simulink model "rfi_blk_v6_casper"  (Simulink 7.1)
settings: Solver=ode45  StartTime=0.0  StopTime=10.0  RelTol=1e-3
undefined symbols (not defined in the file): a, b
libraries: xbsIndex_r4 x13084, xps_library x4
(part 23 of 51)

== system rfi_blk_v6_casper/rfi_blk/mad_comp/Median2/Subsystem5/Median_Accumulator1 ==
  note: Provide reset to the accumulator for clearing after every window.
      | Also this can be used as an enable for calculating median.
      | An interlock logic can be created such that when all the accumulator outputs are zero, median output would be disabled (also lock it with the input enable)
  data_in : Inport  OutDataType=sfix(16)  OutScaling=2^0
  acc_reset : Inport  Port=2  OutDataType=sfix(16)  OutScaling=2^0
  Accumulator{,1..7} (8 alike) : xbsIndex_r4/Accumulator  operation=Add  n_bits=10  overflow=Wrap  scale=1  rst=on  hasbypass=off  en=on  dbl_ovrd=off  use_behavioral_HDL=on  implementation=Fabric  block_type=accum
      settings: infoedit="Adder or subtractor-based accumulator.   Output type and binary point position match the input.<P><P>Hardware notes: When \"Reinitialize with input 'b' on reset\" is selected, the accumulator is forced to run at the system rate even if the input 'b' is running at a slower rate."  block_version=10.1.3
  Constant : xbsIndex_r4/Constant  arith_type=Signed (2's comp)  const=bin_val1  n_bits=8  bin_pt=0  explicit_period=on  period=1  equ=P=C  opselect=C  inp2=PCIN>>17  opr=+  inp1=P  carry=CIN  dbl_ovrd=off  block_type=constant
  Constant1 : xbsIndex_r4/Constant  arith_type=Signed (2's comp)  const=bin_val2  n_bits=8  bin_pt=0  explicit_period=on  period=1  equ=P=C  opselect=C  inp2=PCIN>>17  opr=+  inp1=P  carry=CIN  dbl_ovrd=off  block_type=constant
  Constant2 : xbsIndex_r4/Constant  arith_type=Signed (2's comp)  const=bin_val3  n_bits=8  bin_pt=0  explicit_period=on  period=1  equ=P=C  opselect=C  inp2=PCIN>>17  opr=+  inp1=P  carry=CIN  dbl_ovrd=off  block_type=constant
  Constant3 : xbsIndex_r4/Constant  arith_type=Signed (2's comp)  const=bin_val4  n_bits=8  bin_pt=0  explicit_period=on  period=1  equ=P=C  opselect=C  inp2=PCIN>>17  opr=+  inp1=P  carry=CIN  dbl_ovrd=off  block_type=constant
  Constant4 : xbsIndex_r4/Constant  arith_type=Signed (2's comp)  const=bin_val5  n_bits=8  bin_pt=0  explicit_period=on  period=1  equ=P=C  opselect=C  inp2=PCIN>>17  opr=+  inp1=P  carry=CIN  dbl_ovrd=off  block_type=constant
  Constant5 : xbsIndex_r4/Constant  arith_type=Signed (2's comp)  const=bin_val6  n_bits=8  bin_pt=0  explicit_period=on  period=1  equ=P=C  opselect=C  inp2=PCIN>>17  opr=+  inp1=P  carry=CIN  dbl_ovrd=off  block_type=constant
  Constant6 : xbsIndex_r4/Constant  arith_type=Signed (2's comp)  const=bin_val7  n_bits=8  bin_pt=0  explicit_period=on  period=1  equ=P=C  opselect=C  inp2=PCIN>>17  opr=+  inp1=P  carry=CIN  dbl_ovrd=off  block_type=constant
  Constant7 : xbsIndex_r4/Constant  arith_type=Signed (2's comp)  const=bin_val8  n_bits=8  bin_pt=0  explicit_period=on  period=1  equ=P=C  opselect=C  inp2=PCIN>>17  opr=+  inp1=P  carry=CIN  dbl_ovrd=off  block_type=constant
  Constant8 : xbsIndex_r4/Constant  arith_type=Unsigned  const=1  n_bits=8  bin_pt=0  explicit_period=on  period=1  equ=P=C  opselect=C  inp2=PCIN>>17  opr=+  inp1=P  carry=CIN  dbl_ovrd=off  block_type=constant
  Convert{1..8} (8 alike) : xbsIndex_r4/Convert  arith_type=Boolean  n_bits=16  bin_pt=14  quantization=Truncate  overflow=Wrap  latency=0  dbl_ovrd=off  pipeline=off  block_type=convert
      settings: block_version=10.1.3
  Relational{,1..7} (8 alike) : xbsIndex_r4/Relational  mode=a<=b  en=off  latency=1  dbl_ovrd=off  block_type=relational
      settings: block_version=10.1.3
  Subsystem : Subsystem
  Subsystem1 : Subsystem
  Subsystem2 : Subsystem
  Subsystem3 : Subsystem
  Subsystem4 : Subsystem
  Subsystem5 : Subsystem
  Subsystem6 : Subsystem
  Subsystem7 : Subsystem
  Out1 : Outport
  Out2 : Outport  Port=2
  Out3 : Outport  Port=3
  Out4 : Outport  Port=4
  Out5 : Outport  Port=5
  Out6 : Outport  Port=6
  Out7 : Outport  Port=7
  Out8 : Outport  Port=8
  inc_signal : Outport  Port=9
  wires:
  Constant/1 -> Relational/2
  Constant1/1 -> Relational1/2
  Constant2/1 -> Relational2/2
  Constant4/1 -> Relational4/2
  Constant3/1 -> Relational3/2
  Constant5/1 -> Relational5/2
  Constant6/1 -> Relational6/2
  Constant7/1 -> Relational7/2
  data_in/1 -> Relational/1, Relational1/1, Relational2/1, Relational3/1, Relational4/1, Relational5/1, Relational6/1, Relational7/1
  Constant8/1 -> Accumulator/1, Accumulator1/1, Accumulator2/1, Accumulator3/1, Accumulator4/1, Accumulator5/1, Accumulator6/1, Accumulator7/1
  Relational/1 -> Convert3/1
  Relational1/1 -> Convert1/1
  Relational2/1 -> Convert2/1
  Relational3/1 -> Convert4/1
  Relational4/1 -> Convert5/1
  Relational5/1 -> Convert6/1
  Relational6/1 -> Convert7/1
  Relational7/1 -> Convert8/1
  Convert3/1 -> Accumulator/3
  Convert1/1 -> Accumulator1/3
  Convert2/1 -> Accumulator2/3
  Convert4/1 -> Accumulator3/3
  Convert5/1 -> Accumulator4/3
  Convert6/1 -> Accumulator5/3
  Convert7/1 -> Accumulator6/3
  Convert8/1 -> Accumulator7/3, inc_signal/1
  acc_reset/1 -> Accumulator/2, Accumulator1/2, Accumulator2/2, Accumulator3/2, Accumulator4/2, Accumulator5/2, Accumulator6/2, Accumulator7/2, Subsystem/2(acc_reset_in), Subsystem1/2(acc_reset_in), Subsystem2/2(acc_reset_in), Subsystem3/2(acc_reset_in), Subsystem4/2(acc_reset_in), Subsystem5/2(acc_reset_in), Subsystem6/2(acc_reset_in), Subsystem7/2(acc_reset_in)
  Accumulator/1 -> Subsystem/1(acc_out_in)
  Subsystem/1(adder_out) -> Out1/1
  Accumulator1/1 -> Subsystem1/1(acc_out_in)
  Subsystem1/1(adder_out) -> Out2/1
  Accumulator2/1 -> Subsystem2/1(acc_out_in)
  Subsystem2/1(adder_out) -> Out3/1
  Subsystem3/1(adder_out) -> Out4/1
  Accumulator3/1 -> Subsystem3/1(acc_out_in)
  Accumulator4/1 -> Subsystem4/1(acc_out_in)
  Subsystem4/1(adder_out) -> Out5/1
  Accumulator5/1 -> Subsystem5/1(acc_out_in)
  Subsystem5/1(adder_out) -> Out6/1
  Subsystem6/1(adder_out) -> Out7/1
  Accumulator6/1 -> Subsystem6/1(acc_out_in)
  Accumulator7/1 -> Subsystem7/1(acc_out_in)
  Subsystem7/1(adder_out) -> Out8/1

== system rfi_blk_v6_casper/rfi_blk/mad_comp/Median2/Subsystem5/Median_Accumulator2 ==
  data_in : Inport  OutDataType=sfix(16)  OutScaling=2^0
  acc_reset : Inport  Port=2  OutDataType=sfix(16)  OutScaling=2^0
  Accumulator{,1..7} (8 alike) : xbsIndex_r4/Accumulator  operation=Add  n_bits=10  overflow=Wrap  scale=1  rst=on  hasbypass=off  en=on  dbl_ovrd=off  use_behavioral_HDL=on  implementation=Fabric  block_type=accum
      settings: infoedit="Adder or subtractor-based accumulator.   Output type and binary point position match the input.<P><P>Hardware notes: When \"Reinitialize with input 'b' on reset\" is selected, the accumulator is forced to run at the system rate even if the input 'b' is running at a slower rate."  block_version=10.1.3
  Constant : xbsIndex_r4/Constant  arith_type=Signed (2's comp)  const=bin_val9  n_bits=8  bin_pt=0  explicit_period=on  period=1  equ=P=C  opselect=C  inp2=PCIN>>17  opr=+  inp1=P  carry=CIN  dbl_ovrd=off  block_type=constant
  Constant1 : xbsIndex_r4/Constant  arith_type=Signed (2's comp)  const=bin_val10  n_bits=8  bin_pt=0  explicit_period=on  period=1  equ=P=C  opselect=C  inp2=PCIN>>17  opr=+  inp1=P  carry=CIN  dbl_ovrd=off  block_type=constant
  Constant2 : xbsIndex_r4/Constant  arith_type=Signed (2's comp)  const=bin_val11  n_bits=8  bin_pt=0  explicit_period=on  period=1  equ=P=C  opselect=C  inp2=PCIN>>17  opr=+  inp1=P  carry=CIN  dbl_ovrd=off  block_type=constant
  Constant3 : xbsIndex_r4/Constant  arith_type=Signed (2's comp)  const=bin_val12  n_bits=8  bin_pt=0  explicit_period=on  period=1  equ=P=C  opselect=C  inp2=PCIN>>17  opr=+  inp1=P  carry=CIN  dbl_ovrd=off  block_type=constant
  Constant4 : xbsIndex_r4/Constant  arith_type=Signed (2's comp)  const=bin_val13  n_bits=8  bin_pt=0  explicit_period=on  period=1  equ=P=C  opselect=C  inp2=PCIN>>17  opr=+  inp1=P  carry=CIN  dbl_ovrd=off  block_type=constant
  Constant5 : xbsIndex_r4/Constant  arith_type=Signed (2's comp)  const=bin_val14  n_bits=8  bin_pt=0  explicit_period=on  period=1  equ=P=C  opselect=C  inp2=PCIN>>17  opr=+  inp1=P  carry=CIN  dbl_ovrd=off  block_type=constant
  Constant6 : xbsIndex_r4/Constant  arith_type=Signed (2's comp)  const=bin_val15  n_bits=8  bin_pt=0  explicit_period=on  period=1  equ=P=C  opselect=C  inp2=PCIN>>17  opr=+  inp1=P  carry=CIN  dbl_ovrd=off  block_type=constant
  Constant7 : xbsIndex_r4/Constant  arith_type=Signed (2's comp)  const=bin_val16  n_bits=8  bin_pt=0  explicit_period=on  period=1  equ=P=C  opselect=C  inp2=PCIN>>17  opr=+  inp1=P  carry=CIN  dbl_ovrd=off  block_type=constant
  Constant8 : xbsIndex_r4/Constant  arith_type=Unsigned  const=1  n_bits=8  bin_pt=0  explicit_period=on  period=1  equ=P=C  opselect=C  inp2=PCIN>>17  opr=+  inp1=P  carry=CIN  dbl_ovrd=off  block_type=constant
  Convert{1..8} (8 alike) : xbsIndex_r4/Convert  arith_type=Boolean  n_bits=16  bin_pt=14  quantization=Truncate  overflow=Wrap  latency=0  dbl_ovrd=off  pipeline=off  block_type=convert
      settings: block_version=10.1.3
  Relational{,1..7} (8 alike) : xbsIndex_r4/Relational  mode=a<=b  en=off  latency=1  dbl_ovrd=off  block_type=relational
      settings: block_version=10.1.3
  Subsystem1 : Subsystem
  Subsystem2 : Subsystem
  Subsystem3 : Subsystem
  Subsystem4 : Subsystem
  Subsystem5 : Subsystem
  Subsystem6 : Subsystem
  Subsystem7 : Subsystem
  Subsystem8 : Subsystem
  Out1 : Outport
  Out2 : Outport  Port=2
  Out3 : Outport  Port=3
  Out4 : Outport  Port=4
  Out5 : Outport  Port=5
  Out6 : Outport  Port=6
  Out7 : Outport  Port=7
  Out8 : Outport  Port=8
  inc_signal : Outport  Port=9
  wires:
  Convert8/1 -> Accumulator7/3
  Convert7/1 -> Accumulator6/3
  Convert6/1 -> Accumulator5/3
  Convert5/1 -> Accumulator4/3
  Convert4/1 -> Accumulator3/3
  Convert2/1 -> Accumulator2/3
  Convert1/1 -> Accumulator1/3
  Convert3/1 -> Accumulator/3
  Relational7/1 -> inc_signal/1, Convert8/1
  Relational6/1 -> Convert7/1
  Relational5/1 -> Convert6/1
  Relational4/1 -> Convert5/1
  Relational3/1 -> Convert4/1
  Relational2/1 -> Convert2/1
  Relational1/1 -> Convert1/1
  Constant8/1 -> Accumulator7/1, Accumulator6/1, Accumulator5/1, Accumulator4/1, Accumulator3/1, Accumulator2/1, Accumulator1/1, Accumulator/1
  acc_reset/1 -> Accumulator5/2, Accumulator6/2, Accumulator7/2, Accumulator4/2, Accumulator3/2, Accumulator2/2, Accumulator1/2, Accumulator/2, Subsystem2/2(acc_reset_in), Subsystem3/2(acc_reset_in), Subsystem4/2(acc_reset_in), Subsystem5/2(acc_reset_in), Subsystem6/2(acc_reset_in), Subsystem7/2(acc_reset_in), Subsystem8/2(acc_reset_in), Subsystem1/2(acc_reset_in)
  data_in/1 -> Relational7/1, Relational6/1, Relational5/1, Relational4/1, Relational3/1, Relational2/1, Relational1/1, Relational/1
  Constant7/1 -> Relational7/2
  Constant6/1 -> Relational6/2
  Constant5/1 -> Relational5/2
  Constant3/1 -> Relational3/2
  Constant4/1 -> Relational4/2
  Constant2/1 -> Relational2/2
  Constant1/1 -> Relational1/2
  Constant/1 -> Relational/2
  Relational/1 -> Convert3/1
  Subsystem2/1(adder_out) -> Out1/1
  Accumulator/1 -> Subsystem2/1(acc_out_in)
  Accumulator1/1 -> Subsystem1/1(acc_out_in)
  Subsystem1/1(adder_out) -> Out2/1
  Accumulator2/1 -> Subsystem3/1(acc_out_in)
  Subsystem3/1(adder_out) -> Out3/1
  Subsystem4/1(adder_out) -> Out4/1
  Accumulator3/1 -> Subsystem4/1(acc_out_in)
  Subsystem5/1(adder_out) -> Out5/1
  Accumulator4/1 -> Subsystem5/1(acc_out_in)
  Subsystem6/1(adder_out) -> Out6/1
  Accumulator5/1 -> Subsystem6/1(acc_out_in)
  Subsystem7/1(adder_out) -> Out7/1
  Accumulator6/1 -> Subsystem7/1(acc_out_in)
  Subsystem8/1(adder_out) -> Out8/1
  Accumulator7/1 -> Subsystem8/1(acc_out_in)

== system rfi_blk_v6_casper/rfi_blk/mad_comp/Median2/Subsystem5/Median_Location1 ==
  In1 : Inport  OutDataType=sfix(16)  OutScaling=2^0
  In2 : Inport  Port=2  OutDataType=sfix(16)  OutScaling=2^0
  In3 : Inport  Port=3  OutDataType=sfix(16)  OutScaling=2^0
  In4 : Inport  Port=4  OutDataType=sfix(16)  OutScaling=2^0
  In5 : Inport  Port=5  OutDataType=sfix(16)  OutScaling=2^0
  In6 : Inport  Port=6  OutDataType=sfix(16)  OutScaling=2^0
  In7 : Inport  Port=7  OutDataType=sfix(16)  OutScaling=2^0
  In8 : Inport  Port=8  OutDataType=sfix(16)  OutScaling=2^0
  median_loc : Inport  Port=9  OutDataType=sfix(16)  OutScaling=2^0
  Constant{10,12,14,16,18,20,22,24} (8 alike) : xbsIndex_r4/Constant  arith_type=Boolean  const=1  n_bits=1  bin_pt=0  explicit_period=on  period=1  equ=P=C  opselect=C  inp2=PCIN>>17  opr=+  inp1=P  carry=CIN  dbl_ovrd=off  block_type=constant
  Constant{9,11,13,15,17,19,21,23} (8 alike) : xbsIndex_r4/Constant  arith_type=Boolean  const=0  n_bits=1  bin_pt=0  explicit_period=on  period=1  equ=P=C  opselect=C  inp2=PCIN>>17  opr=+  inp1=P  carry=CIN  dbl_ovrd=off  block_type=constant
  Constant25 : xbsIndex_r4/Constant  arith_type=Signed (2's comp)  const=0  n_bits=8  bin_pt=0  explicit_period=on  period=1  equ=P=C  opselect=C  inp2=PCIN>>17  opr=+  inp1=P  carry=CIN  dbl_ovrd=off  block_type=constant
  Constant26 : xbsIndex_r4/Constant  arith_type=Signed (2's comp)  const=bin_val1  n_bits=8  bin_pt=0  explicit_period=on  period=1  equ=P=C  opselect=C  inp2=PCIN>>17  opr=+  inp1=P  carry=CIN  dbl_ovrd=off  block_type=constant
  Constant27 : xbsIndex_r4/Constant  arith_type=Signed (2's comp)  const=bin_val2  n_bits=8  bin_pt=0  explicit_period=on  period=1  equ=P=C  opselect=C  inp2=PCIN>>17  opr=+  inp1=P  carry=CIN  dbl_ovrd=off  block_type=constant
  Constant28 : xbsIndex_r4/Constant  arith_type=Signed (2's comp)  const=bin_val3  n_bits=8  bin_pt=0  explicit_period=on  period=1  equ=P=C  opselect=C  inp2=PCIN>>17  opr=+  inp1=P  carry=CIN  dbl_ovrd=off  block_type=constant
  Constant29 : xbsIndex_r4/Constant  arith_type=Signed (2's comp)  const=bin_val4  n_bits=8  bin_pt=0  explicit_period=on  period=1  equ=P=C  opselect=C  inp2=PCIN>>17  opr=+  inp1=P  carry=CIN  dbl_ovrd=off  block_type=constant
  Constant30 : xbsIndex_r4/Constant  arith_type=Signed (2's comp)  const=bin_val5  n_bits=8  bin_pt=0  explicit_period=on  period=1  equ=P=C  opselect=C  inp2=PCIN>>17  opr=+  inp1=P  carry=CIN  dbl_ovrd=off  block_type=constant
  Constant31 : xbsIndex_r4/Constant  arith_type=Signed (2's comp)  const=bin_val6  n_bits=8  bin_pt=0  explicit_period=on  period=1  equ=P=C  opselect=C  inp2=PCIN>>17  opr=+  inp1=P  carry=CIN  dbl_ovrd=off  block_type=constant
  Constant32 : xbsIndex_r4/Constant  arith_type=Signed (2's comp)  const=bin_val7  n_bits=8  bin_pt=0  explicit_period=on  period=1  equ=P=C  opselect=C  inp2=PCIN>>17  opr=+  inp1=P  carry=CIN  dbl_ovrd=off  block_type=constant
  Constant33 : xbsIndex_r4/Constant  arith_type=Signed (2's comp)  const=bin_val8  n_bits=8  bin_pt=0  explicit_period=on  period=1  equ=P=C  opselect=C  inp2=PCIN>>17  opr=+  inp1=P  carry=CIN  dbl_ovrd=off  block_type=constant
  Convert : xbsIndex_r4/Convert  arith_type=Boolean  n_bits=16  bin_pt=14  quantization=Truncate  overflow=Wrap  latency=0  dbl_ovrd=off  pipeline=off  block_type=convert
      settings: block_version=10.1.3
  Inverter{,1..6} (7 alike) : xbsIndex_r4/Inverter  en=off  latency=0  dbl_ovrd=off  block_type=inv
  Logical{,2..3,5,7,9,11} (7 alike) : xbsIndex_r4/Logical  logical_function=AND  inputs=2  en=off  latency=0  precision=Full  arith_type=Unsigned  n_bits=16  bin_pt=0  align_bp=on  dbl_ovrd=off  block_type=logical
  Logical1 : xbsIndex_r4/Logical  logical_function=OR  inputs=8  en=off  latency=1  precision=User Defined  arith_type=Unsigned  n_bits=1  bin_pt=0  align_bp=on  dbl_ovrd=off  block_type=logical
  Logical13 : xbsIndex_r4/Logical  logical_function=OR  inputs=8  en=off  latency=1  precision=User Defined  arith_type="Signed  (2's comp)"  n_bits=8  bin_pt=0  align_bp=on  dbl_ovrd=off  block_type=logical
  Mux{,1..7} (8 alike) : xbsIndex_r4/Mux  inputs=2  en=off  latency=0  precision=Full  arith_type="Signed  (2's comp)"  n_bits=8  bin_pt=0  quantization=Truncate  overflow=Wrap  dbl_ovrd=off  block_type=mux
  Mux{8..15} (8 alike) : xbsIndex_r4/Mux  inputs=2  en=off  latency=0  precision=User Defined  arith_type="Signed  (2's comp)"  n_bits=8  bin_pt=0  quantization=Truncate  overflow=Wrap  dbl_ovrd=off  block_type=mux
  Relational{1..8} (8 alike) : xbsIndex_r4/Relational  mode=a>=b  en=off  latency=1  dbl_ovrd=off  block_type=relational
      settings: block_version=10.1.3
  Scope1 : Scope  NumInputPorts=8
      settings: YMin=-5~-5~-5~-5~-5~-5~-5~-5  YMax=5~5~5~5~5~5~5~5  SaveName=ScopeData2
  Scope2 : Scope  NumInputPorts=2
      settings: YMin=-5~-5  YMax=5~5  SaveName=ScopeData3
  Scope3 : Scope  NumInputPorts=2
      settings: YMin=-5~-5  YMax=5~5  SaveName=ScopeData4
  Scope5 : Scope  NumInputPorts=2
      settings: YMin=-5~-5  YMax=5~5  SaveName=ScopeData16
  Out1 : Outport  OutDataType=sfix(16)  OutScaling=2^0
  sel1 : Outport  Port=2  OutDataType=sfix(16)  OutScaling=2^0
  wires:
  median_loc/1 -> Relational1/2, Relational2/2, Relational3/2, Relational4/2, Relational5/2, Relational6/2, Relational7/2, Relational8/2
  Constant9/1 -> Mux/2
  Constant10/1 -> Mux/3
  Relational1/1 -> Mux/1, Inverter/1
  Mux/1 -> [A]
  Inverter/1 -> Logical/1
  Relational2/1 -> Logical/2, Inverter1/1
  Logical/1 -> Mux1/1
  Constant11/1 -> Mux1/2
  Constant12/1 -> Mux1/3
  Relational3/1 -> Logical2/2, Inverter2/1, Scope5/1
  Logical2/1 -> Mux2/1
  Constant13/1 -> Mux2/2
  Constant14/1 -> Mux2/3
  Inverter2/1 -> Logical3/1
  Logical3/1 -> Mux3/1
  Constant15/1 -> Mux3/2
  Constant16/1 -> Mux3/3
  Relational5/1 -> Logical5/2, Inverter4/1, Scope5/2
  Logical5/1 -> Mux4/1
  Constant17/1 -> Mux4/2
  Constant18/1 -> Mux4/3
  Relational6/1 -> Logical7/2, Inverter5/1
  Logical7/1 -> Mux5/1
  Constant19/1 -> Mux5/2
  Constant20/1 -> Mux5/3
  Inverter5/1 -> Logical9/1
  Relational7/1 -> Logical9/2, Inverter6/1
  Constant21/1 -> Mux6/2
  Constant22/1 -> Mux6/3
  Logical9/1 -> Mux6/1
  Inverter6/1 -> Logical11/1
  Relational8/1 -> Logical11/2, Scope3/1
  Constant23/1 -> Mux7/2
  Constant24/1 -> Mux7/3
  Logical11/1 -> Mux7/1
  Mux7/1 -> [H]
  Constant25/1 -> Mux8/2, Mux9/2, Mux10/2, Mux11/2, Mux12/2, Mux13/2, Mux14/2, Mux15/2
  Constant26/1 -> Mux8/3
  Constant27/1 -> Mux9/3
  Constant28/1 -> Mux10/3
  Constant29/1 -> Mux11/3
  Constant30/1 -> Mux12/3
  Constant31/1 -> Mux13/3
  Constant32/1 -> Mux14/3
  Constant33/1 -> Mux15/3
  [A] -> Mux8/1
  [B] -> Mux9/1
  [C] -> Mux10/1
  [D] -> Mux11/1
  [E] -> Mux12/1
  [F] -> Mux13/1
  [G] -> Mux14/1
  [H] -> Mux15/1
  Mux8/1 -> Logical13/1, Scope1/1
  Mux9/1 -> Logical13/2, Scope1/2
  Mux10/1 -> Logical13/3, Scope1/3
  Mux11/1 -> Logical13/4, Scope1/4
  Mux12/1 -> Logical13/5, Scope1/5
  Mux13/1 -> Logical13/6, Scope1/6
  Mux14/1 -> Logical13/7, Scope1/7
  Mux15/1 -> Logical13/8, Scope1/8
  Logical13/1 -> Out1/1
  In1/1 -> Relational1/1
  In2/1 -> Relational2/1
  In3/1 -> Relational3/1
  In4/1 -> Relational4/1
  In5/1 -> Relational5/1
  In6/1 -> Relational6/1
  In7/1 -> Relational7/1
  In8/1 -> Relational8/1, Scope3/2
  Mux1/1 -> [B]
  Mux2/1 -> [C]
  Mux3/1 -> [D]
  Mux4/1 -> [E]
  Mux5/1 -> [F]
  Mux6/1 -> [G]
  Inverter1/1 -> Logical2/1
  Relational4/1 -> Logical3/2, Inverter3/1
  Inverter3/1 -> Logical5/1
  Inverter4/1 -> Logical7/1
  [A] -> Logical1/1
  [B] -> Logical1/2
  [C] -> Logical1/3
  [D] -> Logical1/4
  [E] -> Logical1/5
  [F] -> Logical1/6
  [G] -> Logical1/7
  [H] -> Logical1/8
  Logical1/1 -> Convert/1
  Convert/1 -> sel1/1

== system rfi_blk_v6_casper/rfi_blk/mad_comp/Median2/Subsystem5/Median_Location2 ==
  In1 : Inport  OutDataType=sfix(16)  OutScaling=2^0
  In2 : Inport  Port=2  OutDataType=sfix(16)  OutScaling=2^0
  In3 : Inport  Port=3  OutDataType=sfix(16)  OutScaling=2^0
  In4 : Inport  Port=4  OutDataType=sfix(16)  OutScaling=2^0
  In5 : Inport  Port=5  OutDataType=sfix(16)  OutScaling=2^0
  In6 : Inport  Port=6  OutDataType=sfix(16)  OutScaling=2^0
  In7 : Inport  Port=7  OutDataType=sfix(16)  OutScaling=2^0
  In8 : Inport  Port=8  OutDataType=sfix(16)  OutScaling=2^0
  median_loc : Inport  Port=9  OutDataType=sfix(16)  OutScaling=2^0
  Constant{10,12,14,16,18,20,22,24} (8 alike) : xbsIndex_r4/Constant  arith_type=Boolean  const=1  n_bits=1  bin_pt=0  explicit_period=on  period=1  equ=P=C  opselect=C  inp2=PCIN>>17  opr=+  inp1=P  carry=CIN  dbl_ovrd=off  block_type=constant
  Constant{9,11,13,15,17,19,21,23} (8 alike) : xbsIndex_r4/Constant  arith_type=Boolean  const=0  n_bits=1  bin_pt=0  explicit_period=on  period=1  equ=P=C  opselect=C  inp2=PCIN>>17  opr=+  inp1=P  carry=CIN  dbl_ovrd=off  block_type=constant
  Constant25 : xbsIndex_r4/Constant  arith_type=Signed (2's comp)  const=0  n_bits=8  bin_pt=0  explicit_period=on  period=1  equ=P=C  opselect=C  inp2=PCIN>>17  opr=+  inp1=P  carry=CIN  dbl_ovrd=off  block_type=constant
  Constant26 : xbsIndex_r4/Constant  arith_type=Signed (2's comp)  const=bin_val9  n_bits=8  bin_pt=0  explicit_period=on  period=1  equ=P=C  opselect=C  inp2=PCIN>>17  opr=+  inp1=P  carry=CIN  dbl_ovrd=off  block_type=constant
  Constant27 : xbsIndex_r4/Constant  arith_type=Signed (2's comp)  const=bin_val10  n_bits=8  bin_pt=0  explicit_period=on  period=1  equ=P=C  opselect=C  inp2=PCIN>>17  opr=+  inp1=P  carry=CIN  dbl_ovrd=off  block_type=constant
  Constant28 : xbsIndex_r4/Constant  arith_type=Signed (2's comp)  const=bin_val11  n_bits=8  bin_pt=0  explicit_period=on  period=1  equ=P=C  opselect=C  inp2=PCIN>>17  opr=+  inp1=P  carry=CIN  dbl_ovrd=off  block_type=constant
  Constant29 : xbsIndex_r4/Constant  arith_type=Signed (2's comp)  const=bin_val12  n_bits=8  bin_pt=0  explicit_period=on  period=1  equ=P=C  opselect=C  inp2=PCIN>>17  opr=+  inp1=P  carry=CIN  dbl_ovrd=off  block_type=constant
  Constant30 : xbsIndex_r4/Constant  arith_type=Signed (2's comp)  const=bin_val13  n_bits=8  bin_pt=0  explicit_period=on  period=1  equ=P=C  opselect=C  inp2=PCIN>>17  opr=+  inp1=P  carry=CIN  dbl_ovrd=off  block_type=constant
  Constant31 : xbsIndex_r4/Constant  arith_type=Signed (2's comp)  const=bin_val14  n_bits=8  bin_pt=0  explicit_period=on  period=1  equ=P=C  opselect=C  inp2=PCIN>>17  opr=+  inp1=P  carry=CIN  dbl_ovrd=off  block_type=constant
  Constant32 : xbsIndex_r4/Constant  arith_type=Signed (2's comp)  const=bin_val15  n_bits=8  bin_pt=0  explicit_period=on  period=1  equ=P=C  opselect=C  inp2=PCIN>>17  opr=+  inp1=P  carry=CIN  dbl_ovrd=off  block_type=constant
  Constant33 : xbsIndex_r4/Constant  arith_type=Signed (2's comp)  const=bin_val16  n_bits=8  bin_pt=0  explicit_period=on  period=1  equ=P=C  opselect=C  inp2=PCIN>>17  opr=+  inp1=P  carry=CIN  dbl_ovrd=off  block_type=constant
  Convert{,1..8} (9 alike) : xbsIndex_r4/Convert  arith_type=Boolean  n_bits=16  bin_pt=14  quantization=Truncate  overflow=Wrap  latency=0  dbl_ovrd=off  pipeline=off  block_type=convert
      settings: block_version=10.1.3
  Inverter{,1..6} (7 alike) : xbsIndex_r4/Inverter  en=off  latency=0  dbl_ovrd=off  block_type=inv
  Logical{,2..3,5,7,9,11} (7 alike) : xbsIndex_r4/Logical  logical_function=AND  inputs=2  en=off  latency=0  precision=Full  arith_type=Unsigned  n_bits=16  bin_pt=0  align_bp=on  dbl_ovrd=off  block_type=logical
  Logical1 : xbsIndex_r4/Logical  logical_function=OR  inputs=8  en=off  latency=1  precision=User Defined  arith_type=Unsigned  n_bits=1  bin_pt=0  align_bp=on  dbl_ovrd=off  block_type=logical
  Logical13 : xbsIndex_r4/Logical  logical_function=OR  inputs=8  en=off  latency=1  precision=User Defined  arith_type="Signed  (2's comp)"  n_bits=8  bin_pt=0  align_bp=on  dbl_ovrd=off  block_type=logical
  Mux{,1..7} (8 alike) : xbsIndex_r4/Mux  inputs=2  en=off  latency=0  precision=Full  arith_type=Unsigned  n_bits=16  bin_pt=14  quantization=Truncate  overflow=Wrap  dbl_ovrd=off  block_type=mux
  Mux{8..15} (8 alike) : xbsIndex_r4/Mux  inputs=2  en=off  latency=0  precision=User Defined  arith_type="Signed  (2's comp)"  n_bits=8  bin_pt=0  quantization=Truncate  overflow=Wrap  dbl_ovrd=off  block_type=mux
  Relational{1..8} (8 alike) : xbsIndex_r4/Relational  mode=a>=b  en=off  latency=1  dbl_ovrd=off  block_type=relational
      settings: block_version=10.1.3
  Scope1 : Scope  NumInputPorts=8
      settings: YMin=-5~-5~-5~-5~-5~-5~-5~-5  YMax=5~5~5~5~5~5~5~5  SaveName=ScopeData2
  Out3 : Outport  OutDataType=sfix(16)  OutScaling=2^0
  sel2 : Outport  Port=2  OutDataType=sfix(16)  OutScaling=2^0
  wires:
  Inverter4/1 -> Logical7/1
  Inverter3/1 -> Logical5/1
  Relational4/1 -> Inverter3/1, Logical3/2
  Inverter1/1 -> Logical2/1
  Mux6/1 -> [G]
  Mux5/1 -> [F]
  Mux4/1 -> [E]
  Mux3/1 -> [D]
  Mux2/1 -> [C]
  Mux1/1 -> [B]
  In8/1 -> Relational8/1
  In7/1 -> Relational7/1
  In6/1 -> Relational6/1
  In5/1 -> Relational5/1
  In4/1 -> Relational4/1
  In3/1 -> Relational3/1
  In2/1 -> Relational2/1
  In1/1 -> Relational1/1
  Mux15/1 -> Scope1/8, Logical13/8
  Mux14/1 -> Scope1/7, Logical13/7
  Mux13/1 -> Scope1/6, Logical13/6
  Mux12/1 -> Scope1/5, Logical13/5
  Mux11/1 -> Scope1/4, Logical13/4
  Mux10/1 -> Scope1/3, Logical13/3
  Mux9/1 -> Scope1/2, Logical13/2
  Mux8/1 -> Scope1/1, Logical13/1
  [H] -> Mux15/1
  [G] -> Mux14/1
  [F] -> Mux13/1
  [E] -> Mux12/1
  [D] -> Mux11/1
  [C] -> Mux10/1
  [B] -> Mux9/1
  [A] -> Mux8/1
  Constant33/1 -> Mux15/3
  Constant32/1 -> Mux14/3
  Constant31/1 -> Mux13/3
  Constant30/1 -> Mux12/3
  Constant29/1 -> Mux11/3
  Constant28/1 -> Mux10/3
  Constant27/1 -> Mux9/3
  Constant26/1 -> Mux8/3
  Constant25/1 -> Mux15/2, Mux14/2, Mux13/2, Mux12/2, Mux11/2, Mux10/2, Mux9/2, Mux8/2
  Mux7/1 -> [H]
  Logical11/1 -> Mux7/1
  Constant24/1 -> Mux7/3
  Constant23/1 -> Mux7/2
  Relational8/1 -> Logical11/2
  Inverter6/1 -> Logical11/1
  Logical9/1 -> Mux6/1
  Constant22/1 -> Mux6/3
  Constant21/1 -> Mux6/2
  Relational7/1 -> Inverter6/1, Logical9/2
  Inverter5/1 -> Logical9/1
  Constant20/1 -> Mux5/3
  Constant19/1 -> Mux5/2
  Logical7/1 -> Mux5/1
  Relational6/1 -> Inverter5/1, Logical7/2
  Constant18/1 -> Mux4/3
  Constant17/1 -> Mux4/2
  Logical5/1 -> Mux4/1
  Relational5/1 -> Inverter4/1, Logical5/2
  Constant16/1 -> Mux3/3
  Constant15/1 -> Mux3/2
  Logical3/1 -> Mux3/1
  Inverter2/1 -> Logical3/1
  Constant14/1 -> Mux2/3
  Constant13/1 -> Mux2/2
  Logical2/1 -> Mux2/1
  Relational3/1 -> Inverter2/1, Logical2/2
  Constant12/1 -> Mux1/3
  Constant11/1 -> Mux1/2
  Logical/1 -> Mux1/1
  Relational2/1 -> Inverter1/1, Logical/2
  Inverter/1 -> Logical/1
  Mux/1 -> [A]
  Relational1/1 -> Inverter/1, Mux/1
  Constant10/1 -> Mux/3
  Constant9/1 -> Mux/2
  median_loc/1 -> Relational8/2, Relational7/2, Relational6/2, Relational5/2, Relational4/2, Relational3/2, Relational2/2, Relational1/2
  [A] -> Convert1/1
  [B] -> Convert2/1
  [C] -> Convert3/1
  [D] -> Convert4/1
  [E] -> Convert5/1
  [F] -> Convert6/1
  [G] -> Convert7/1
  [H] -> Convert8/1
  Logical1/1 -> Convert/1
  Convert/1 -> sel2/1
  Convert1/1 -> Logical1/1
  Convert2/1 -> Logical1/2
  Convert3/1 -> Logical1/3
  Convert4/1 -> Logical1/4
  Convert5/1 -> Logical1/5
  Convert6/1 -> Logical1/6
  Convert7/1 -> Logical1/7
  Convert8/1 -> Logical1/8
  Logical13/1 -> Out3/1

== system rfi_blk_v6_casper/rfi_blk/mad_comp/Median2/Subsystem6/Median_Accumulator1 ==
  note: Provide reset to the accumulator for clearing after every window.
      | Also this can be used as an enable for calculating median.
      | An interlock logic can be created such that when all the accumulator outputs are zero, median output would be disabled (also lock it with the input enable)
  data_in : Inport  OutDataType=sfix(16)  OutScaling=2^0
  acc_reset : Inport  Port=2  OutDataType=sfix(16)  OutScaling=2^0
  Accumulator{,1..7} (8 alike) : xbsIndex_r4/Accumulator  operation=Add  n_bits=10  overflow=Wrap  scale=1  rst=on  hasbypass=off  en=on  dbl_ovrd=off  use_behavioral_HDL=on  implementation=Fabric  block_type=accum
      settings: infoedit="Adder or subtractor-based accumulator.   Output type and binary point position match the input.<P><P>Hardware notes: When \"Reinitialize with input 'b' on reset\" is selected, the accumulator is forced to run at the system rate even if the input 'b' is running at a slower rate."  block_version=10.1.3
  Constant : xbsIndex_r4/Constant  arith_type=Signed (2's comp)  const=bin_val1  n_bits=8  bin_pt=0  explicit_period=on  period=1  equ=P=C  opselect=C  inp2=PCIN>>17  opr=+  inp1=P  carry=CIN  dbl_ovrd=off  block_type=constant
  Constant1 : xbsIndex_r4/Constant  arith_type=Signed (2's comp)  const=bin_val2  n_bits=8  bin_pt=0  explicit_period=on  period=1  equ=P=C  opselect=C  inp2=PCIN>>17  opr=+  inp1=P  carry=CIN  dbl_ovrd=off  block_type=constant
  Constant2 : xbsIndex_r4/Constant  arith_type=Signed (2's comp)  const=bin_val3  n_bits=8  bin_pt=0  explicit_period=on  period=1  equ=P=C  opselect=C  inp2=PCIN>>17  opr=+  inp1=P  carry=CIN  dbl_ovrd=off  block_type=constant
  Constant3 : xbsIndex_r4/Constant  arith_type=Signed (2's comp)  const=bin_val4  n_bits=8  bin_pt=0  explicit_period=on  period=1  equ=P=C  opselect=C  inp2=PCIN>>17  opr=+  inp1=P  carry=CIN  dbl_ovrd=off  block_type=constant
  Constant4 : xbsIndex_r4/Constant  arith_type=Signed (2's comp)  const=bin_val5  n_bits=8  bin_pt=0  explicit_period=on  period=1  equ=P=C  opselect=C  inp2=PCIN>>17  opr=+  inp1=P  carry=CIN  dbl_ovrd=off  block_type=constant
  Constant5 : xbsIndex_r4/Constant  arith_type=Signed (2's comp)  const=bin_val6  n_bits=8  bin_pt=0  explicit_period=on  period=1  equ=P=C  opselect=C  inp2=PCIN>>17  opr=+  inp1=P  carry=CIN  dbl_ovrd=off  block_type=constant
  Constant6 : xbsIndex_r4/Constant  arith_type=Signed (2's comp)  const=bin_val7  n_bits=8  bin_pt=0  explicit_period=on  period=1  equ=P=C  opselect=C  inp2=PCIN>>17  opr=+  inp1=P  carry=CIN  dbl_ovrd=off  block_type=constant
  Constant7 : xbsIndex_r4/Constant  arith_type=Signed (2's comp)  const=bin_val8  n_bits=8  bin_pt=0  explicit_period=on  period=1  equ=P=C  opselect=C  inp2=PCIN>>17  opr=+  inp1=P  carry=CIN  dbl_ovrd=off  block_type=constant
  Constant8 : xbsIndex_r4/Constant  arith_type=Unsigned  const=1  n_bits=8  bin_pt=0  explicit_period=on  period=1  equ=P=C  opselect=C  inp2=PCIN>>17  opr=+  inp1=P  carry=CIN  dbl_ovrd=off  block_type=constant
  Convert{1..8} (8 alike) : xbsIndex_r4/Convert  arith_type=Boolean  n_bits=16  bin_pt=14  quantization=Truncate  overflow=Wrap  latency=0  dbl_ovrd=off  pipeline=off  block_type=convert
      settings: block_version=10.1.3
  Relational{,1..7} (8 alike) : xbsIndex_r4/Relational  mode=a<=b  en=off  latency=1  dbl_ovrd=off  block_type=relational
      settings: block_version=10.1.3
  Subsystem : Subsystem
  Subsystem1 : Subsystem
  Subsystem2 : Subsystem
  Subsystem3 : Subsystem
  Subsystem4 : Subsystem
  Subsystem5 : Subsystem
  Subsystem6 : Subsystem
  Subsystem7 : Subsystem
  Out1 : Outport
  Out2 : Outport  Port=2
  Out3 : Outport  Port=3
  Out4 : Outport  Port=4
  Out5 : Outport  Port=5
  Out6 : Outport  Port=6
  Out7 : Outport  Port=7
  Out8 : Outport  Port=8
  inc_signal : Outport  Port=9
  wires:
  Constant/1 -> Relational/2
  Constant1/1 -> Relational1/2
  Constant2/1 -> Relational2/2
  Constant4/1 -> Relational4/2
  Constant3/1 -> Relational3/2
  Constant5/1 -> Relational5/2
  Constant6/1 -> Relational6/2
  Constant7/1 -> Relational7/2
  data_in/1 -> Relational/1, Relational1/1, Relational2/1, Relational3/1, Relational4/1, Relational5/1, Relational6/1, Relational7/1
  Constant8/1 -> Accumulator/1, Accumulator1/1, Accumulator2/1, Accumulator3/1, Accumulator4/1, Accumulator5/1, Accumulator6/1, Accumulator7/1
  Relational/1 -> Convert3/1
  Relational1/1 -> Convert1/1
  Relational2/1 -> Convert2/1
  Relational3/1 -> Convert4/1
  Relational4/1 -> Convert5/1
  Relational5/1 -> Convert6/1
  Relational6/1 -> Convert7/1
  Relational7/1 -> Convert8/1
  Convert3/1 -> Accumulator/3
  Convert1/1 -> Accumulator1/3
  Convert2/1 -> Accumulator2/3
  Convert4/1 -> Accumulator3/3
  Convert5/1 -> Accumulator4/3
  Convert6/1 -> Accumulator5/3
  Convert7/1 -> Accumulator6/3
  Convert8/1 -> Accumulator7/3, inc_signal/1
  acc_reset/1 -> Accumulator/2, Accumulator1/2, Accumulator2/2, Accumulator3/2, Accumulator4/2, Accumulator5/2, Accumulator6/2, Accumulator7/2, Subsystem/2(acc_reset_in), Subsystem1/2(acc_reset_in), Subsystem2/2(acc_reset_in), Subsystem3/2(acc_reset_in), Subsystem4/2(acc_reset_in), Subsystem5/2(acc_reset_in), Subsystem6/2(acc_reset_in), Subsystem7/2(acc_reset_in)
  Accumulator/1 -> Subsystem/1(acc_out_in)
  Subsystem/1(adder_out) -> Out1/1
  Accumulator1/1 -> Subsystem1/1(acc_out_in)
  Subsystem1/1(adder_out) -> Out2/1
  Accumulator2/1 -> Subsystem2/1(acc_out_in)
  Subsystem2/1(adder_out) -> Out3/1
  Subsystem3/1(adder_out) -> Out4/1
  Accumulator3/1 -> Subsystem3/1(acc_out_in)
  Accumulator4/1 -> Subsystem4/1(acc_out_in)
  Subsystem4/1(adder_out) -> Out5/1
  Accumulator5/1 -> Subsystem5/1(acc_out_in)
  Subsystem5/1(adder_out) -> Out6/1
  Subsystem6/1(adder_out) -> Out7/1
  Accumulator6/1 -> Subsystem6/1(acc_out_in)
  Accumulator7/1 -> Subsystem7/1(acc_out_in)
  Subsystem7/1(adder_out) -> Out8/1

== system rfi_blk_v6_casper/rfi_blk/mad_comp/Median2/Subsystem6/Median_Accumulator2 ==
  data_in : Inport  OutDataType=sfix(16)  OutScaling=2^0
  acc_reset : Inport  Port=2  OutDataType=sfix(16)  OutScaling=2^0
  Accumulator{,1..7} (8 alike) : xbsIndex_r4/Accumulator  operation=Add  n_bits=10  overflow=Wrap  scale=1  rst=on  hasbypass=off  en=on  dbl_ovrd=off  use_behavioral_HDL=on  implementation=Fabric  block_type=accum
      settings: infoedit="Adder or subtractor-based accumulator.   Output type and binary point position match the input.<P><P>Hardware notes: When \"Reinitialize with input 'b' on reset\" is selected, the accumulator is forced to run at the system rate even if the input 'b' is running at a slower rate."  block_version=10.1.3
  Constant : xbsIndex_r4/Constant  arith_type=Signed (2's comp)  const=bin_val9  n_bits=8  bin_pt=0  explicit_period=on  period=1  equ=P=C  opselect=C  inp2=PCIN>>17  opr=+  inp1=P  carry=CIN  dbl_ovrd=off  block_type=constant
  Constant1 : xbsIndex_r4/Constant  arith_type=Signed (2's comp)  const=bin_val10  n_bits=8  bin_pt=0  explicit_period=on  period=1  equ=P=C  opselect=C  inp2=PCIN>>17  opr=+  inp1=P  carry=CIN  dbl_ovrd=off  block_type=constant
  Constant2 : xbsIndex_r4/Constant  arith_type=Signed (2's comp)  const=bin_val11  n_bits=8  bin_pt=0  explicit_period=on  period=1  equ=P=C  opselect=C  inp2=PCIN>>17  opr=+  inp1=P  carry=CIN  dbl_ovrd=off  block_type=constant
  Constant3 : xbsIndex_r4/Constant  arith_type=Signed (2's comp)  const=bin_val12  n_bits=8  bin_pt=0  explicit_period=on  period=1  equ=P=C  opselect=C  inp2=PCIN>>17  opr=+  inp1=P  carry=CIN  dbl_ovrd=off  block_type=constant
  Constant4 : xbsIndex_r4/Constant  arith_type=Signed (2's comp)  const=bin_val13  n_bits=8  bin_pt=0  explicit_period=on  period=1  equ=P=C  opselect=C  inp2=PCIN>>17  opr=+  inp1=P  carry=CIN  dbl_ovrd=off  block_type=constant
  Constant5 : xbsIndex_r4/Constant  arith_type=Signed (2's comp)  const=bin_val14  n_bits=8  bin_pt=0  explicit_period=on  period=1  equ=P=C  opselect=C  inp2=PCIN>>17  opr=+  inp1=P  carry=CIN  dbl_ovrd=off  block_type=constant
  Constant6 : xbsIndex_r4/Constant  arith_type=Signed (2's comp)  const=bin_val15  n_bits=8  bin_pt=0  explicit_period=on  period=1  equ=P=C  opselect=C  inp2=PCIN>>17  opr=+  inp1=P  carry=CIN  dbl_ovrd=off  block_type=constant
  Constant7 : xbsIndex_r4/Constant  arith_type=Signed (2's comp)  const=bin_val16  n_bits=8  bin_pt=0  explicit_period=on  period=1  equ=P=C  opselect=C  inp2=PCIN>>17  opr=+  inp1=P  carry=CIN  dbl_ovrd=off  block_type=constant
  Constant8 : xbsIndex_r4/Constant  arith_type=Unsigned  const=1  n_bits=8  bin_pt=0  explicit_period=on  period=1  equ=P=C  opselect=C  inp2=PCIN>>17  opr=+  inp1=P  carry=CIN  dbl_ovrd=off  block_type=constant
  Convert{1..8} (8 alike) : xbsIndex_r4/Convert  arith_type=Boolean  n_bits=16  bin_pt=14  quantization=Truncate  overflow=Wrap  latency=0  dbl_ovrd=off  pipeline=off  block_type=convert
      settings: block_version=10.1.3
  Relational{,1..7} (8 alike) : xbsIndex_r4/Relational  mode=a<=b  en=off  latency=1  dbl_ovrd=off  block_type=relational
      settings: block_version=10.1.3
  Subsystem1 : Subsystem
  Subsystem2 : Subsystem
  Subsystem3 : Subsystem
  Subsystem4 : Subsystem
  Subsystem5 : Subsystem
  Subsystem6 : Subsystem
  Subsystem7 : Subsystem
  Subsystem8 : Subsystem
  Out1 : Outport
  Out2 : Outport  Port=2
  Out3 : Outport  Port=3
  Out4 : Outport  Port=4
  Out5 : Outport  Port=5
  Out6 : Outport  Port=6
  Out7 : Outport  Port=7
  Out8 : Outport  Port=8
  inc_signal : Outport  Port=9
  wires:
  Convert8/1 -> Accumulator7/3
  Convert7/1 -> Accumulator6/3
  Convert6/1 -> Accumulator5/3
  Convert5/1 -> Accumulator4/3
  Convert4/1 -> Accumulator3/3
  Convert2/1 -> Accumulator2/3
  Convert1/1 -> Accumulator1/3
  Convert3/1 -> Accumulator/3
  Relational7/1 -> inc_signal/1, Convert8/1
  Relational6/1 -> Convert7/1
  Relational5/1 -> Convert6/1
  Relational4/1 -> Convert5/1
  Relational3/1 -> Convert4/1
  Relational2/1 -> Convert2/1
  Relational1/1 -> Convert1/1
  Constant8/1 -> Accumulator7/1, Accumulator6/1, Accumulator5/1, Accumulator4/1, Accumulator3/1, Accumulator2/1, Accumulator1/1, Accumulator/1
  acc_reset/1 -> Accumulator5/2, Accumulator6/2, Accumulator7/2, Accumulator4/2, Accumulator3/2, Accumulator2/2, Accumulator1/2, Accumulator/2, Subsystem2/2(acc_reset_in), Subsystem3/2(acc_reset_in), Subsystem4/2(acc_reset_in), Subsystem5/2(acc_reset_in), Subsystem6/2(acc_reset_in), Subsystem7/2(acc_reset_in), Subsystem8/2(acc_reset_in), Subsystem1/2(acc_reset_in)
  data_in/1 -> Relational7/1, Relational6/1, Relational5/1, Relational4/1, Relational3/1, Relational2/1, Relational1/1, Relational/1
  Constant7/1 -> Relational7/2
  Constant6/1 -> Relational6/2
  Constant5/1 -> Relational5/2
  Constant3/1 -> Relational3/2
  Constant4/1 -> Relational4/2
  Constant2/1 -> Relational2/2
  Constant1/1 -> Relational1/2
  Constant/1 -> Relational/2
  Relational/1 -> Convert3/1
  Subsystem2/1(adder_out) -> Out1/1
  Accumulator/1 -> Subsystem2/1(acc_out_in)
  Accumulator1/1 -> Subsystem1/1(acc_out_in)
  Subsystem1/1(adder_out) -> Out2/1
  Accumulator2/1 -> Subsystem3/1(acc_out_in)
  Subsystem3/1(adder_out) -> Out3/1
  Subsystem4/1(adder_out) -> Out4/1
  Accumulator3/1 -> Subsystem4/1(acc_out_in)
  Subsystem5/1(adder_out) -> Out5/1
  Accumulator4/1 -> Subsystem5/1(acc_out_in)
  Subsystem6/1(adder_out) -> Out6/1
  Accumulator5/1 -> Subsystem6/1(acc_out_in)
  Subsystem7/1(adder_out) -> Out7/1
  Accumulator6/1 -> Subsystem7/1(acc_out_in)
  Subsystem8/1(adder_out) -> Out8/1
  Accumulator7/1 -> Subsystem8/1(acc_out_in)

== system rfi_blk_v6_casper/rfi_blk/mad_comp/Median2/Subsystem6/Median_Location1 ==
  In1 : Inport  OutDataType=sfix(16)  OutScaling=2^0
  In2 : Inport  Port=2  OutDataType=sfix(16)  OutScaling=2^0
  In3 : Inport  Port=3  OutDataType=sfix(16)  OutScaling=2^0
  In4 : Inport  Port=4  OutDataType=sfix(16)  OutScaling=2^0
  In5 : Inport  Port=5  OutDataType=sfix(16)  OutScaling=2^0
  In6 : Inport  Port=6  OutDataType=sfix(16)  OutScaling=2^0
  In7 : Inport  Port=7  OutDataType=sfix(16)  OutScaling=2^0
  In8 : Inport  Port=8  OutDataType=sfix(16)  OutScaling=2^0
  median_loc : Inport  Port=9  OutDataType=sfix(16)  OutScaling=2^0
  Constant{10,12,14,16,18,20,22,24} (8 alike) : xbsIndex_r4/Constant  arith_type=Boolean  const=1  n_bits=1  bin_pt=0  explicit_period=on  period=1  equ=P=C  opselect=C  inp2=PCIN>>17  opr=+  inp1=P  carry=CIN  dbl_ovrd=off  block_type=constant
  Constant{9,11,13,15,17,19,21,23} (8 alike) : xbsIndex_r4/Constant  arith_type=Boolean  const=0  n_bits=1  bin_pt=0  explicit_period=on  period=1  equ=P=C  opselect=C  inp2=PCIN>>17  opr=+  inp1=P  carry=CIN  dbl_ovrd=off  block_type=constant
  Constant25 : xbsIndex_r4/Constant  arith_type=Signed (2's comp)  const=0  n_bits=8  bin_pt=0  explicit_period=on  period=1  equ=P=C  opselect=C  inp2=PCIN>>17  opr=+  inp1=P  carry=CIN  dbl_ovrd=off  block_type=constant
  Constant26 : xbsIndex_r4/Constant  arith_type=Signed (2's comp)  const=bin_val1  n_bits=8  bin_pt=0  explicit_period=on  period=1  equ=P=C  opselect=C  inp2=PCIN>>17  opr=+  inp1=P  carry=CIN  dbl_ovrd=off  block_type=constant
  Constant27 : xbsIndex_r4/Constant  arith_type=Signed (2's comp)  const=bin_val2  n_bits=8  bin_pt=0  explicit_period=on  period=1  equ=P=C  opselect=C  inp2=PCIN>>17  opr=+  inp1=P  carry=CIN  dbl_ovrd=off  block_type=constant
  Constant28 : xbsIndex_r4/Constant  arith_type=Signed (2's comp)  const=bin_val3  n_bits=8  bin_pt=0  explicit_period=on  period=1  equ=P=C  opselect=C  inp2=PCIN>>17  opr=+  inp1=P  carry=CIN  dbl_ovrd=off  block_type=constant
  Constant29 : xbsIndex_r4/Constant  arith_type=Signed (2's comp)  const=bin_val4  n_bits=8  bin_pt=0  explicit_period=on  period=1  equ=P=C  opselect=C  inp2=PCIN>>17  opr=+  inp1=P  carry=CIN  dbl_ovrd=off  block_type=constant
  Constant30 : xbsIndex_r4/Constant  arith_type=Signed (2's comp)  const=bin_val5  n_bits=8  bin_pt=0  explicit_period=on  period=1  equ=P=C  opselect=C  inp2=PCIN>>17  opr=+  inp1=P  carry=CIN  dbl_ovrd=off  block_type=constant
  Constant31 : xbsIndex_r4/Constant  arith_type=Signed (2's comp)  const=bin_val6  n_bits=8  bin_pt=0  explicit_period=on  period=1  equ=P=C  opselect=C  inp2=PCIN>>17  opr=+  inp1=P  carry=CIN  dbl_ovrd=off  block_type=constant
  Constant32 : xbsIndex_r4/Constant  arith_type=Signed (2's comp)  const=bin_val7  n_bits=8  bin_pt=0  explicit_period=on  period=1  equ=P=C  opselect=C  inp2=PCIN>>17  opr=+  inp1=P  carry=CIN  dbl_ovrd=off  block_type=constant
  Constant33 : xbsIndex_r4/Constant  arith_type=Signed (2's comp)  const=bin_val8  n_bits=8  bin_pt=0  explicit_period=on  period=1  equ=P=C  opselect=C  inp2=PCIN>>17  opr=+  inp1=P  carry=CIN  dbl_ovrd=off  block_type=constant
  Convert : xbsIndex_r4/Convert  arith_type=Boolean  n_bits=16  bin_pt=14  quantization=Truncate  overflow=Wrap  latency=0  dbl_ovrd=off  pipeline=off  block_type=convert
      settings: block_version=10.1.3
  Inverter{,1..6} (7 alike) : xbsIndex_r4/Inverter  en=off  latency=0  dbl_ovrd=off  block_type=inv
  Logical{,2..3,5,7,9,11} (7 alike) : xbsIndex_r4/Logical  logical_function=AND  inputs=2  en=off  latency=0  precision=Full  arith_type=Unsigned  n_bits=16  bin_pt=0  align_bp=on  dbl_ovrd=off  block_type=logical
  Logical1 : xbsIndex_r4/Logical  logical_function=OR  inputs=8  en=off  latency=1  precision=User Defined  arith_type=Unsigned  n_bits=1  bin_pt=0  align_bp=on  dbl_ovrd=off  block_type=logical
  Logical13 : xbsIndex_r4/Logical  logical_function=OR  inputs=8  en=off  latency=1  precision=User Defined  arith_type="Signed  (2's comp)"  n_bits=8  bin_pt=0  align_bp=on  dbl_ovrd=off  block_type=logical
  Mux{,1..7} (8 alike) : xbsIndex_r4/Mux  inputs=2  en=off  latency=0  precision=Full  arith_type="Signed  (2's comp)"  n_bits=8  bin_pt=0  quantization=Truncate  overflow=Wrap  dbl_ovrd=off  block_type=mux
  Mux{8..15} (8 alike) : xbsIndex_r4/Mux  inputs=2  en=off  latency=0  precision=User Defined  arith_type="Signed  (2's comp)"  n_bits=8  bin_pt=0  quantization=Truncate  overflow=Wrap  dbl_ovrd=off  block_type=mux
  Relational{1..8} (8 alike) : xbsIndex_r4/Relational  mode=a>=b  en=off  latency=1  dbl_ovrd=off  block_type=relational
      settings: block_version=10.1.3
  Scope1 : Scope  NumInputPorts=8
      settings: YMin=-5~-5~-5~-5~-5~-5~-5~-5  YMax=5~5~5~5~5~5~5~5  SaveName=ScopeData2
  Scope2 : Scope  NumInputPorts=2
      settings: YMin=-5~-5  YMax=5~5  SaveName=ScopeData3
  Scope3 : Scope  NumInputPorts=2
      settings: YMin=-5~-5  YMax=5~5  SaveName=ScopeData4
  Scope5 : Scope  NumInputPorts=2
      settings: YMin=-5~-5  YMax=5~5  SaveName=ScopeData16
  Out1 : Outport  OutDataType=sfix(16)  OutScaling=2^0
  sel1 : Outport  Port=2  OutDataType=sfix(16)  OutScaling=2^0
  wires:
  median_loc/1 -> Relational1/2, Relational2/2, Relational3/2, Relational4/2, Relational5/2, Relational6/2, Relational7/2, Relational8/2
  Constant9/1 -> Mux/2
  Constant10/1 -> Mux/3
  Relational1/1 -> Mux/1, Inverter/1
  Mux/1 -> [A]
  Inverter/1 -> Logical/1
  Relational2/1 -> Logical/2, Inverter1/1
  Logical/1 -> Mux1/1
  Constant11/1 -> Mux1/2
  Constant12/1 -> Mux1/3
  Relational3/1 -> Logical2/2, Inverter2/1, Scope5/1
  Logical2/1 -> Mux2/1
  Constant13/1 -> Mux2/2
  Constant14/1 -> Mux2/3
  Inverter2/1 -> Logical3/1
  Logical3/1 -> Mux3/1
  Constant15/1 -> Mux3/2
  Constant16/1 -> Mux3/3
  Relational5/1 -> Logical5/2, Inverter4/1, Scope5/2
  Logical5/1 -> Mux4/1
  Constant17/1 -> Mux4/2
  Constant18/1 -> Mux4/3
  Relational6/1 -> Logical7/2, Inverter5/1
  Logical7/1 -> Mux5/1
  Constant19/1 -> Mux5/2
  Constant20/1 -> Mux5/3
  Inverter5/1 -> Logical9/1
  Relational7/1 -> Logical9/2, Inverter6/1
  Constant21/1 -> Mux6/2
  Constant22/1 -> Mux6/3
  Logical9/1 -> Mux6/1
  Inverter6/1 -> Logical11/1
  Relational8/1 -> Logical11/2, Scope3/1
  Constant23/1 -> Mux7/2
  Constant24/1 -> Mux7/3
  Logical11/1 -> Mux7/1
  Mux7/1 -> [H]
  Constant25/1 -> Mux8/2, Mux9/2, Mux10/2, Mux11/2, Mux12/2, Mux13/2, Mux14/2, Mux15/2
  Constant26/1 -> Mux8/3
  Constant27/1 -> Mux9/3
  Constant28/1 -> Mux10/3
  Constant29/1 -> Mux11/3
  Constant30/1 -> Mux12/3
  Constant31/1 -> Mux13/3
  Constant32/1 -> Mux14/3
  Constant33/1 -> Mux15/3
  [A] -> Mux8/1
  [B] -> Mux9/1
  [C] -> Mux10/1
  [D] -> Mux11/1
  [E] -> Mux12/1
  [F] -> Mux13/1
  [G] -> Mux14/1
  [H] -> Mux15/1
  Mux8/1 -> Logical13/1, Scope1/1
  Mux9/1 -> Logical13/2, Scope1/2
  Mux10/1 -> Logical13/3, Scope1/3
  Mux11/1 -> Logical13/4, Scope1/4
  Mux12/1 -> Logical13/5, Scope1/5
  Mux13/1 -> Logical13/6, Scope1/6
  Mux14/1 -> Logical13/7, Scope1/7
  Mux15/1 -> Logical13/8, Scope1/8
  Logical13/1 -> Out1/1
  In1/1 -> Relational1/1
  In2/1 -> Relational2/1
  In3/1 -> Relational3/1
  In4/1 -> Relational4/1
  In5/1 -> Relational5/1
  In6/1 -> Relational6/1
  In7/1 -> Relational7/1
  In8/1 -> Relational8/1, Scope3/2
  Mux1/1 -> [B]
  Mux2/1 -> [C]
  Mux3/1 -> [D]
  Mux4/1 -> [E]
  Mux5/1 -> [F]
  Mux6/1 -> [G]
  Inverter1/1 -> Logical2/1
  Relational4/1 -> Logical3/2, Inverter3/1
  Inverter3/1 -> Logical5/1
  Inverter4/1 -> Logical7/1
  [A] -> Logical1/1
  [B] -> Logical1/2
  [C] -> Logical1/3
  [D] -> Logical1/4
  [E] -> Logical1/5
  [F] -> Logical1/6
  [G] -> Logical1/7
  [H] -> Logical1/8
  Logical1/1 -> Convert/1
  Convert/1 -> sel1/1
